simulink model "ofdm_txrx_mimo"  (Simulink 7.1)
settings: Solver=ode45  StartTime=0.0  StopTime=6e3  RelTol=1e-3
PreLoadFcn:
  | ofdm_rx_mimo_init
InitFcn:
  | ofdm_rx_mimo_init
StartFcn:
  | ofdm_rx_mimo_init
values from neighbouring files (symbols this model uses):
  BER_RAM_init_size = 2048    [ofdm_tx_mimo_init.m]
  CPLength = 16    [ofdm_tx_mimo_init.m]
  CRCPolynomial = hex2dec('04c11db7')    [ofdm_tx_mimo_init.m]
  freqOffset_track_filtCoef_i = hex2dec('2500')/2^32    [ofdm_rx_mimo_init.m]
  freqOffset_track_filtCoef_p = hex2dec('d0000')/2^32    [ofdm_rx_mimo_init.m]
  hi = zeros(1,length(cci))    [ofdm_rx_mimo_init.m]
  hr = zeros(1,length(ccr))    [ofdm_rx_mimo_init.m]
  max_CP_length = 16    [ofdm_tx_mimo_init.m]
  max_num_baseRateSymbols = 31    [ofdm_tx_mimo_init.m]
  max_num_subcarriers = 64    [ofdm_tx_mimo_init.m]
  max_num_trainingSymbols = 15    [ofdm_tx_mimo_init.m]
  max_numBytes = 16384    [ofdm_tx_mimo_init.m]
  max_OFDM_symbols = 2047    [ofdm_tx_mimo_init.m]
  modConstellation_qam16 = .75*[1 3 -1 -3]./3    [ofdm_tx_mimo_init.m]
  modConstellation_qam256 = 0.9375*[3 1 5 7 11 9 13 15 -3 -1 -5 -7 -11 -9 -13 -15]./15    [ofdm_tx_mimo_init.m]
  modConstellation_qam64 = 0.875*[3 1 5 7 -3 -1 -5 -7]./7    [ofdm_tx_mimo_init.m]
  modConstellation_qpsk = [1 -1]./sqrt(2)    [ofdm_tx_mimo_init.m]
  numBaseRateSymbols = 2    [ofdm_tx_mimo_init.m]
  numSubcarriers = 64    [ofdm_tx_mimo_init.m]
  numTrainingSymbols = 2    [ofdm_tx_mimo_init.m]
  pilot1_indicies = 7 + ( (64-7) * 2^16)    (= 3735559)    [ofdm_tx_mimo_init.m]
  pilot2_indicies = 21 + ( (64-21) * 2^16)    (= 2818069)    [ofdm_tx_mimo_init.m]
  pilotValue_neg = hex2dec('8000') + (2^16 * 0)    [ofdm_tx_mimo_init.m]
  pilotValue_pos = hex2dec('7FFF') + (2^16 * 0)    [ofdm_tx_mimo_init.m]
  pkt_crossCorr_thresh = 0.7    [ofdm_rx_mimo_init.m]
  pkt_energy_thresh = 0    [ofdm_rx_mimo_init.m]
  pkt_numPayloadBytes = 1400+42-6    (= 1436)    [ofdm_tx_mimo_init.m]
  pktByteNums = numHeaderBytes +  				byteIndex_numPayloadBytes(1)*2^8 +  				byteIndex_numPayloadBytes(2)*2^16 +  				byteIndex_simpleDynModMasks * 2^24    [ofdm_rx_mimo_init.m]
  PktDet_Delay = 26 + Symbol_Timing_Offset*2^7    (= 1050)    [ofdm_rx_mimo_init.m]
  preamble = 6*[0 shortsyms_10 longsyms_2]    [ofdm_tx_mimo_init.m]
  preamble_I = real(preamble)    [ofdm_tx_mimo_init.m]
  preamble_Q = imag(preamble)    [ofdm_tx_mimo_init.m]
  RAM_init_size = max_numBytes    (= 16384)    [ofdm_tx_mimo_init.m]
  reg_Interrupt_PktBuf_Control = reg_InterruptControl +  	1 * 2^16 +  	0 * 2^24    (= 65656)    [ofdm_rx_mimo_init.m]
  rx_controlBits = 1 * 2  		 + 1 * 4  		 + 0 * 8  		 + 1 * 16 * rx_SISO_Mode  		 + 1 * 32  		 + 1 * 64  		 + 0 * 128  		 + 1 * 256  		 + 0 * 512  		 + 1 * 1024  		 + 0 * 2048  		 + 1 * 4096  		 + 1 * 8192  		 + 0 * 16384  		 + 1 * 65536  		 + 1 * 131072  		 + 0 * 262144  		 + 1 * 524288    [ofdm_rx_mimo_init.m]
  Rx_PhaseNoiseTrack_K = 0.6094    [ofdm_rx_mimo_init.m]
  rx_postEq_scaling = round(rxScaling*2^11) * (1 + 2^16)    [ofdm_rx_mimo_init.m]
  RxDataScrambling_Seq = [40 198 78 63 82 173 102 245 48 111 172 115 147 230 216 93 72 65 62 2 205 242 122 90 128 83 105 97 73 10 5 252]    [ofdm_tx_mimo_init.m]
  simOnly_numSamples = length(preamble)+( (numSubcarriers+CPLength)*(numTrainingSymbols + numBaseRateSymbols + numFullRateSymbols/2) )    [ofdm_tx_mimo_init.m]
  train = [train train]    [ofdm_tx_mimo_init.m]
  tx_controlBits = (antB_preambleShift * 2^4) + (2^0 * tx_SISO_Mode) + 4*0 + 8*1    (= 73)    [ofdm_tx_mimo_init.m]
  TxDataScrambling_Seq = [40 198 78 63 82 173 102 245 48 111 172 115 147 230 216 93 72 65 62 2 205 242 122 90 128 83 105 97 73 10 5 252]    [ofdm_tx_mimo_init.m]
  txReg_symbolCounts = (2^16 * numFullRateSymbols) + (2^8 *numBaseRateSymbols ) + numTrainingSymbols    [ofdm_tx_mimo_init.m]
  TxRx_FFTScaling = bin2dec('010111') + (bin2dec('000101') * 2^6)    [ofdm_tx_mimo_init.m]
undefined symbols (not defined in the file or in its folder): AntA, AntB, BaseRate, CRC_table_gen, gcbh, intfilt, pktTiming_count_payloadStart, pktTiming_symbol_length, xlBlockLoadCallback, xlGetSimulinkPeriod
libraries: xbsIndex_r4 x4212, dspmlti4 x4, dspsigops x4, commchan3 x2, simulink x2, commrflib2 x2, dspsrcs4 x1
(part 8 of 43)

== system ofdm_txrx_mimo/Memory-mapped Registers/Rx Registers/Read-Write Register2 ==
  Constant : xbsIndex_r4/Constant  arith_type=Boolean  const=1  n_bits=16  bin_pt=14  explicit_period=on  period=sampPeriod  equ=P=C  opselect=C  inp2=PCIN>>17  opr=+  inp1=P  carry=CIN  dbl_ovrd=off  block_type=constant
      settings: block_version=8.2.02
  From Register : xbsIndex_r4/From Register  shared_memory_name='Rx_pktByteNums_w'  init=131864  period=sampPeriod  ownership=Locally owned and initialized  arith_type=Unsigned  n_bits=32  bin_pt=0  dbl_ovrd=off  block_type=fromreg
      settings: AttributesFormatString=From Register\n<< %<shared_memory_name> >>\n%<n_bits>\n%<period>\n%<bin_pt>\n%<arith_type>\n%<ownership>\n%<init>  infoedit="Register block that reads data to a shared memory register.  Delay of one sample period."  block_version=8.2.02
  Terminator : Terminator
  To Register : xbsIndex_r4/To Register  shared_memory_name='Rx_pktByteNums_r'  init=131864  ownership=Locally owned and initialized  explicit_data_type=on  arith_type=Unsigned  n_bits=32  bin_pt=0  dbl_ovrd=off  block_type=toreg
      settings: AttributesFormatString=To Register\n<< %<shared_memory_name> >>  infoedit="Register block that writes data to a shared memory register.  Delay of one sample period."  block_version=8.2.02
  D : Outport  OutDataType=sfix(16)  OutScaling=2^0
  wires:
  To Register/1 -> Terminator/1
  Constant/1 -> To Register/2
  From Register/1 -> To Register/1, D/1

== system ofdm_txrx_mimo/Memory-mapped Registers/Rx Registers/Read-Write Register3 ==
  Constant : xbsIndex_r4/Constant  arith_type=Boolean  const=1  n_bits=16  bin_pt=14  explicit_period=on  period=sampPeriod  equ=P=C  opselect=C  inp2=PCIN>>17  opr=+  inp1=P  carry=CIN  dbl_ovrd=off  block_type=constant
      settings: block_version=8.2.02
  From Register : xbsIndex_r4/From Register  shared_memory_name='Rx_PktDet_Delay_w'  init=1050  period=sampPeriod  ownership=Locally owned and initialized  arith_type=Unsigned  n_bits=32  bin_pt=0  dbl_ovrd=off  block_type=fromreg
      settings: AttributesFormatString=From Register\n<< %<shared_memory_name> >>\n%<n_bits>\n%<period>\n%<bin_pt>\n%<arith_type>\n%<ownership>\n%<init>  infoedit="Register block that reads data to a shared memory register.  Delay of one sample period."  block_version=8.2.02
  Terminator : Terminator
  To Register : xbsIndex_r4/To Register  shared_memory_name='Rx_PktDet_Delay_r'  init=1050  ownership=Locally owned and initialized  explicit_data_type=on  arith_type=Unsigned  n_bits=32  bin_pt=0  dbl_ovrd=off  block_type=toreg
      settings: AttributesFormatString=To Register\n<< %<shared_memory_name> >>  infoedit="Register block that writes data to a shared memory register.  Delay of one sample period."  block_version=8.2.02
  D : Outport  OutDataType=sfix(16)  OutScaling=2^0
  wires:
  To Register/1 -> Terminator/1
  Constant/1 -> To Register/2
  From Register/1 -> To Register/1, D/1

== system ofdm_txrx_mimo/Memory-mapped Registers/Rx Registers/Read-Write Register4 ==
  Constant : xbsIndex_r4/Constant  arith_type=Boolean  const=1  n_bits=16  bin_pt=14  explicit_period=on  period=sampPeriod  equ=P=C  opselect=C  inp2=PCIN>>17  opr=+  inp1=P  carry=CIN  dbl_ovrd=off  block_type=constant
      settings: block_version=8.2.02
  From Register : xbsIndex_r4/From Register  shared_memory_name='Rx_OFDM_SymbolCounts_w'  init=131074  period=sampPeriod  ownership=Locally owned and initialized  arith_type=Unsigned  n_bits=32  bin_pt=0  dbl_ovrd=off  block_type=fromreg
      settings: AttributesFormatString=From Register\n<< %<shared_memory_name> >>\n%<n_bits>\n%<period>\n%<bin_pt>\n%<arith_type>\n%<ownership>\n%<init>  infoedit="Register block that reads data to a shared memory register.  Delay of one sample period."  block_version=8.2.02
  Terminator : Terminator
  To Register : xbsIndex_r4/To Register  shared_memory_name='Rx_OFDM_SymbolCounts_r'  init=131074  ownership=Locally owned and initialized  explicit_data_type=on  arith_type=Unsigned  n_bits=32  bin_pt=0  dbl_ovrd=off  block_type=toreg
      settings: AttributesFormatString=To Register\n<< %<shared_memory_name> >>  infoedit="Register block that writes data to a shared memory register.  Delay of one sample period."  block_version=8.2.02
  D : Outport  OutDataType=sfix(16)  OutScaling=2^0
  wires:
  To Register/1 -> Terminator/1
  Constant/1 -> To Register/2
  From Register/1 -> To Register/1, D/1

== system ofdm_txrx_mimo/Memory-mapped Registers/Rx Registers/Read-Write Register5 ==
  Constant : xbsIndex_r4/Constant  arith_type=Boolean  const=1  n_bits=16  bin_pt=14  explicit_period=on  period=sampPeriod  equ=P=C  opselect=C  inp2=PCIN>>17  opr=+  inp1=P  carry=CIN  dbl_ovrd=off  block_type=constant
      settings: block_version=8.2.02
  From Register : xbsIndex_r4/From Register  shared_memory_name='Rx_ControlBits_w'  init=734582  period=sampPeriod  ownership=Locally owned and initialized  arith_type=Unsigned  n_bits=32  bin_pt=0  dbl_ovrd=off  block_type=fromreg
      settings: AttributesFormatString=From Register\n<< %<shared_memory_name> >>\n%<n_bits>\n%<period>\n%<bin_pt>\n%<arith_type>\n%<ownership>\n%<init>  infoedit="Register block that reads data to a shared memory register.  Delay of one sample period."  block_version=8.2.02
  Terminator : Terminator
  To Register : xbsIndex_r4/To Register  shared_memory_name='Rx_ControlBits_r'  init=734582  ownership=Locally owned and initialized  explicit_data_type=on  arith_type=Unsigned  n_bits=32  bin_pt=0  dbl_ovrd=off  block_type=toreg
      settings: AttributesFormatString=To Register\n<< %<shared_memory_name> >>  infoedit="Register block that writes data to a shared memory register.  Delay of one sample period."  block_version=8.2.02
  D : Outport  OutDataType=sfix(16)  OutScaling=2^0
  wires:
  From Register/1 -> D/1, To Register/1
  Constant/1 -> To Register/2
  To Register/1 -> Terminator/1

== system ofdm_txrx_mimo/Memory-mapped Registers/Rx Registers/Write-Only Register ==
  From Register : xbsIndex_r4/From Register  shared_memory_name='Rx_pktDet_Tresholds_w'  init=0.7  period=sampPeriod  ownership=Locally owned and initialized  arith_type=Unsigned  n_bits=32  bin_pt=0  dbl_ovrd=off  block_type=fromreg
      settings: AttributesFormatString=From Register\n<< %<shared_memory_name> >>\n%<n_bits>\n%<period>\n%<bin_pt>\n%<arith_type>\n%<ownership>\n%<init>  infoedit="Register block that reads data to a shared memory register.  Delay of one sample period."  block_version=8.2.02
  D : Outport  OutDataType=sfix(16)  OutScaling=2^0
  wires:
  From Register/1 -> D/1

== system ofdm_txrx_mimo/Memory-mapped Registers/Rx Registers/Write-Only Register1 ==
  From Register : xbsIndex_r4/From Register  shared_memory_name='Rx_Constellation_Scaling_w'  init=268439552  period=sampPeriod  ownership=Locally owned and initialized  arith_type=Unsigned  n_bits=32  bin_pt=0  dbl_ovrd=off  block_type=fromreg
      settings: AttributesFormatString=From Register\n<< %<shared_memory_name> >>\n%<n_bits>\n%<period>\n%<bin_pt>\n%<arith_type>\n%<ownership>\n%<init>  infoedit="Register block that reads data to a shared memory register.  Delay of one sample period."  block_version=8.2.02
  D : Outport  OutDataType=sfix(16)  OutScaling=2^0
  wires:
  From Register/1 -> D/1

== system ofdm_txrx_mimo/Memory-mapped Registers/Rx Registers/Write-Only Register2 ==
  From Register : xbsIndex_r4/From Register  shared_memory_name='Rx_FreqOffFilt_B_KI_w'  init=9472  period=sampPeriod  ownership=Locally owned and initialized  arith_type=Unsigned  n_bits=32  bin_pt=0  dbl_ovrd=off  block_type=fromreg
      settings: AttributesFormatString=From Register\n<< %<shared_memory_name> >>\n%<n_bits>\n%<period>\n%<bin_pt>\n%<arith_type>\n%<ownership>\n%<init>  infoedit="Register block that reads data to a shared memory register.  Delay of one sample period."  block_version=8.2.02
  D : Outport  OutDataType=sfix(16)  OutScaling=2^0
  wires:
  From Register/1 -> D/1

== system ofdm_txrx_mimo/Memory-mapped Registers/Rx Registers/Write-Only Register3 ==
  From Register : xbsIndex_r4/From Register  shared_memory_name='Rx_FreqOffFilt_B_KP_w'  init=851968  period=sampPeriod  ownership=Locally owned and initialized  arith_type=Unsigned  n_bits=32  bin_pt=0  dbl_ovrd=off  block_type=fromreg
      settings: AttributesFormatString=From Register\n<< %<shared_memory_name> >>\n%<n_bits>\n%<period>\n%<bin_pt>\n%<arith_type>\n%<ownership>\n%<init>  infoedit="Register block that reads data to a shared memory register.  Delay of one sample period."  block_version=8.2.02
  D : Outport  OutDataType=sfix(16)  OutScaling=2^0
  wires:
  From Register/1 -> D/1

== system ofdm_txrx_mimo/Memory-mapped Registers/Rx Registers/Write-Only Register4 ==
  From Register : xbsIndex_r4/From Register  shared_memory_name='Rx_PhaseNoiseTrack_K_w'  init=40896141.7216  period=sampPeriod  ownership=Locally owned and initialized  arith_type=Unsigned  n_bits=32  bin_pt=0  dbl_ovrd=off  block_type=fromreg
      settings: AttributesFormatString=From Register\n<< %<shared_memory_name> >>\n%<n_bits>\n%<period>\n%<bin_pt>\n%<arith_type>\n%<ownership>\n%<init>  infoedit="Register block that reads data to a shared memory register.  Delay of one sample period."  block_version=8.2.02
  D : Outport  OutDataType=sfix(16)  OutScaling=2^0
  wires:
  From Register/1 -> D/1

== system ofdm_txrx_mimo/Memory-mapped Registers/Rx Registers/Write-Only Register5 ==
  From Register : xbsIndex_r4/From Register  shared_memory_name='Rx_EVM_w'  init=20  period=sampPeriod  ownership=Locally owned and initialized  arith_type=Unsigned  n_bits=7  bin_pt=0  dbl_ovrd=off  block_type=fromreg
      settings: AttributesFormatString=From Register\n<< %<shared_memory_name> >>\n%<n_bits>\n%<period>\n%<bin_pt>\n%<arith_type>\n%<ownership>\n%<init>  infoedit="Register block that reads data to a shared memory register.  Delay of one sample period."  block_version=8.2.02
  D : Outport  OutDataType=sfix(16)  OutScaling=2^0
  wires:
  From Register/1 -> D/1

== system ofdm_txrx_mimo/Memory-mapped Registers/Tx Registers/Read Only Register ==
  D : Inport  OutDataType=sfix(16)  OutScaling=2^0
  En : Inport  Port=2  OutDataType=sfix(16)  OutScaling=2^0
  Convert : xbsIndex_r4/Convert  arith_type=Unsigned  n_bits=32  bin_pt=0  quantization=Truncate  overflow=Wrap  latency=0  dbl_ovrd=off  pipeline=off  block_type=convert
      settings: block_version=10.1
  Reinterpret : xbsIndex_r4/Reinterpret  force_arith_type=on  arith_type=Unsigned  force_bin_pt=on  bin_pt=0  block_type=reinterpret
      settings: infoedit="Changes signal type without altering the binary representation.   You can changed the signal between signed and unsigned, and relocate the binary point.<P><P>Hardware notes: In hardware this block costs nothing.<P><P>Example:  Suppose the input is 6 bits wide, signed, with 2 fractional bits, and the output is forced to unsigned with 0 fractional bits.  Then an input of -2.0 (1110.00 in binary 2's complement) becomes an output of 56 (111000 in binary)."  block_version=10.1
  Terminator : Terminator
  To Register : xbsIndex_r4/To Register  shared_memory_name='Tx_PktRunning'  init=0  ownership=Locally owned and initialized  explicit_data_type=on  arith_type=Unsigned  n_bits=32  bin_pt=0  dbl_ovrd=off  block_type=toreg
      settings: AttributesFormatString=To Register\n<< %<shared_memory_name> >>  infoedit="Register block that writes data to a shared memory register.  Delay of one sample period."  block_version=8.2.02
  wires:
  En/1 -> To Register/2
  To Register/1 -> Terminator/1
  D/1 -> Reinterpret/1
  Reinterpret/1 -> Convert/1
  Convert/1 -> To Register/1

== system ofdm_txrx_mimo/Memory-mapped Registers/Tx Registers/Read-Write Register ==
  Constant : xbsIndex_r4/Constant  arith_type=Boolean  const=1  n_bits=16  bin_pt=14  explicit_period=on  period=sampPeriod  equ=P=C  opselect=C  inp2=PCIN>>17  opr=+  inp1=P  carry=CIN  dbl_ovrd=off  block_type=constant
      settings: block_version=8.2.02
  From Register : xbsIndex_r4/From Register  shared_memory_name='Tx_Start_Reset_Control_w'  init=0  period=sampPeriod  ownership=Locally owned and initialized  arith_type=Unsigned  n_bits=32  bin_pt=0  dbl_ovrd=off  block_type=fromreg
      settings: AttributesFormatString=From Register\n<< %<shared_memory_name> >>\n%<n_bits>\n%<period>\n%<bin_pt>\n%<arith_type>\n%<ownership>\n%<init>  infoedit="Register block that reads data to a shared memory register.  Delay of one sample period."  block_version=8.2.02
  Terminator : Terminator
  To Register : xbsIndex_r4/To Register  shared_memory_name='Tx_Start_Reset_Control_r'  init=0  ownership=Locally owned and initialized  explicit_data_type=on  arith_type=Unsigned  n_bits=32  bin_pt=0  dbl_ovrd=off  block_type=toreg
      settings: AttributesFormatString=To Register\n<< %<shared_memory_name> >>  infoedit="Register block that writes data to a shared memory register.  Delay of one sample period."  block_version=8.2.02
  D : Outport  OutDataType=sfix(16)  OutScaling=2^0
  wires:
  To Register/1 -> Terminator/1
  Constant/1 -> To Register/2
  From Register/1 -> To Register/1, D/1

== system ofdm_txrx_mimo/Memory-mapped Registers/Tx Registers/Read-Write Register1 ==
  Constant : xbsIndex_r4/Constant  arith_type=Boolean  const=1  n_bits=16  bin_pt=14  explicit_period=on  period=sampPeriod  equ=P=C  opselect=C  inp2=PCIN>>17  opr=+  inp1=P  carry=CIN  dbl_ovrd=off  block_type=constant
      settings: block_version=8.2.02
  From Register : xbsIndex_r4/From Register  shared_memory_name='Tx_ControlBits_w'  init=73  period=sampPeriod  ownership=Locally owned and initialized  arith_type=Unsigned  n_bits=32  bin_pt=0  dbl_ovrd=off  block_type=fromreg
      settings: AttributesFormatString=From Register\n<< %<shared_memory_name> >>\n%<n_bits>\n%<period>\n%<bin_pt>\n%<arith_type>\n%<ownership>\n%<init>  infoedit="Register block that reads data to a shared memory register.  Delay of one sample period."  block_version=8.2.02
  Terminator : Terminator
  To Register : xbsIndex_r4/To Register  shared_memory_name='Tx_ControlBits_r'  init=73  ownership=Locally owned and initialized  explicit_data_type=on  arith_type=Unsigned  n_bits=32  bin_pt=0  dbl_ovrd=off  block_type=toreg
      settings: AttributesFormatString=To Register\n<< %<shared_memory_name> >>  infoedit="Register block that writes data to a shared memory register.  Delay of one sample period."  block_version=8.2.02
  D : Outport  OutDataType=sfix(16)  OutScaling=2^0
  wires:
  To Register/1 -> Terminator/1
  Constant/1 -> To Register/2
  From Register/1 -> To Register/1, D/1

== system ofdm_txrx_mimo/Memory-mapped Registers/Tx Registers/Read-Write Register2 ==
  Constant : xbsIndex_r4/Constant  arith_type=Boolean  const=1  n_bits=16  bin_pt=14  explicit_period=on  period=sampPeriod  equ=P=C  opselect=C  inp2=PCIN>>17  opr=+  inp1=P  carry=CIN  dbl_ovrd=off  block_type=constant
      settings: block_version=8.2.02
  From Register : xbsIndex_r4/From Register  shared_memory_name='Tx_OFDM_SymCounts_w'  init=15729154  period=sampPeriod  ownership=Locally owned and initialized  arith_type=Unsigned  n_bits=32  bin_pt=0  dbl_ovrd=off  block_type=fromreg
      settings: AttributesFormatString=From Register\n<< %<shared_memory_name> >>\n%<n_bits>\n%<period>\n%<bin_pt>\n%<arith_type>\n%<ownership>\n%<init>  infoedit="Register block that reads data to a shared memory register.  Delay of one sample period."  block_version=8.2.02
  Terminator : Terminator
  To Register : xbsIndex_r4/To Register  shared_memory_name='Tx_OFDM_SymCounts_r'  init=15729154  ownership=Locally owned and initialized  explicit_data_type=on  arith_type=Unsigned  n_bits=32  bin_pt=0  dbl_ovrd=off  block_type=toreg
      settings: AttributesFormatString=To Register\n<< %<shared_memory_name> >>  infoedit="Register block that writes data to a shared memory register.  Delay of one sample period."  block_version=8.2.02
  D : Outport  OutDataType=sfix(16)  OutScaling=2^0
  wires:
  To Register/1 -> Terminator/1
  Constant/1 -> To Register/2
  From Register/1 -> To Register/1, D/1

== system ofdm_txrx_mimo/Memory-mapped Registers/Tx Registers/Write-Only Register ==
  From Register : xbsIndex_r4/From Register  shared_memory_name='Tx_PreambleMasking_w'  init=0  period=sampPeriod  ownership=Locally owned and initialized  arith_type=Unsigned  n_bits=32  bin_pt=0  dbl_ovrd=off  block_type=fromreg
      settings: AttributesFormatString=From Register\n<< %<shared_memory_name> >>\n%<n_bits>\n%<period>\n%<bin_pt>\n%<arith_type>\n%<ownership>\n%<init>  infoedit="Register block that reads data to a shared memory register.  Delay of one sample period."  block_version=8.2.02
  D : Outport  OutDataType=sfix(16)  OutScaling=2^0
  wires:
  From Register/1 -> D/1

== system ofdm_txrx_mimo/Memory-mapped Registers/Tx Registers/Write-Only Register1 ==
  From Register : xbsIndex_r4/From Register  shared_memory_name='Tx_Scaling_w'  init=1768954592  period=sampPeriod  ownership=Locally owned and initialized  arith_type=Unsigned  n_bits=32  bin_pt=0  dbl_ovrd=off  block_type=fromreg
      settings: AttributesFormatString=From Register\n<< %<shared_memory_name> >>\n%<n_bits>\n%<period>\n%<bin_pt>\n%<arith_type>\n%<ownership>\n%<init>  infoedit="Register block that reads data to a shared memory register.  Delay of one sample period."  block_version=8.2.02
  D : Outport  OutDataType=sfix(16)  OutScaling=2^0
  wires:
  From Register/1 -> D/1

== system ofdm_txrx_mimo/Memory-mapped Registers/Tx Registers/Write-Only Register2 ==
  From Register : xbsIndex_r4/From Register  shared_memory_name='Tx_Pilots_Index1_w'  init=3735559  period=sampPeriod  ownership=Locally owned and initialized  arith_type=Unsigned  n_bits=32  bin_pt=0  dbl_ovrd=off  block_type=fromreg
      settings: AttributesFormatString=From Register\n<< %<shared_memory_name> >>\n%<n_bits>\n%<period>\n%<bin_pt>\n%<arith_type>\n%<ownership>\n%<init>  infoedit="Register block that reads data to a shared memory register.  Delay of one sample period."  block_version=8.2.02
  D : Outport  OutDataType=sfix(16)  OutScaling=2^0
  wires:
  From Register/1 -> D/1

== system ofdm_txrx_mimo/Memory-mapped Registers/Tx Registers/Write-Only Register3 ==
  From Register : xbsIndex_r4/From Register  shared_memory_name='Tx_Pilots_Index2_w'  init=2818069  period=sampPeriod  ownership=Locally owned and initialized  arith_type=Unsigned  n_bits=32  bin_pt=0  dbl_ovrd=off  block_type=fromreg
      settings: AttributesFormatString=From Register\n<< %<shared_memory_name> >>\n%<n_bits>\n%<period>\n%<bin_pt>\n%<arith_type>\n%<ownership>\n%<init>  infoedit="Register block that reads data to a shared memory register.  Delay of one sample period."  block_version=8.2.02
  D : Outport  OutDataType=sfix(16)  OutScaling=2^0
  wires:
  From Register/1 -> D/1

== system ofdm_txrx_mimo/Memory-mapped Registers/Tx Registers/Write-Only Register4 ==
  From Register : xbsIndex_r4/From Register  shared_memory_name='Tx_Pilots_Value1_w'  init=32768  period=sampPeriod  ownership=Locally owned and initialized  arith_type=Unsigned  n_bits=32  bin_pt=0  dbl_ovrd=off  block_type=fromreg
      settings: AttributesFormatString=From Register\n<< %<shared_memory_name> >>\n%<n_bits>\n%<period>\n%<bin_pt>\n%<arith_type>\n%<ownership>\n%<init>  infoedit="Register block that reads data to a shared memory register.  Delay of one sample period."  block_version=8.2.02
  D : Outport  OutDataType=sfix(16)  OutScaling=2^0
  wires:
  From Register/1 -> D/1

== system ofdm_txrx_mimo/Memory-mapped Registers/Tx Registers/Write-Only Register5 ==
  From Register : xbsIndex_r4/From Register  shared_memory_name='Tx_Pilots_Value2_w'  init=32767  period=sampPeriod  ownership=Locally owned and initialized  arith_type=Unsigned  n_bits=32  bin_pt=0  dbl_ovrd=off  block_type=fromreg
      settings: AttributesFormatString=From Register\n<< %<shared_memory_name> >>\n%<n_bits>\n%<period>\n%<bin_pt>\n%<arith_type>\n%<ownership>\n%<init>  infoedit="Register block that reads data to a shared memory register.  Delay of one sample period."  block_version=8.2.02
  D : Outport  OutDataType=sfix(16)  OutScaling=2^0
  wires:
  From Register/1 -> D/1

== system ofdm_txrx_mimo/Memory-mapped Registers/TxRx Registers/InterruptControl ==
  Reg_8b : Inport  OutDataType=sfix(16)  OutScaling=2^0
  LSB+0 : xbsIndex_r4/Slice  nbits=1  boolean_output=on  mode=Lower Bit Location + Width  bit1=0  base1=MSB of Input  bit0=0  base0=LSB of Input  dbl_ovrd=off  block_type=slice
      settings: infoedit="Extracts a given range of bits from each input sample and presents it at the output.  The output type is ordinarily unsigned with binary point at zero, but can be Boolean when the slice is one bit wide.<P><P>Hardware notes: In hardware this block costs nothing."  block_version=VER_STRING_GOES_HERE
  LSB+1 : xbsIndex_r4/Slice  nbits=1  boolean_output=on  mode=Lower Bit Location + Width  bit1=0  base1=MSB of Input  bit0=1  base0=LSB of Input  dbl_ovrd=off  block_type=slice
      settings: infoedit="Extracts a given range of bits from each input sample and presents it at the output.  The output type is ordinarily unsigned with binary point at zero, but can be Boolean when the slice is one bit wide.<P><P>Hardware notes: In hardware this block costs nothing."  block_version=VER_STRING_GOES_HERE
  LSB+2 : xbsIndex_r4/Slice  nbits=1  boolean_output=off  mode=Lower Bit Location + Width  bit1=0  base1=MSB of Input  bit0=2  base0=LSB of Input  dbl_ovrd=off  block_type=slice
      settings: infoedit="Extracts a given range of bits from each input sample and presents it at the output.  The output type is ordinarily unsigned with binary point at zero, but can be Boolean when the slice is one bit wide.<P><P>Hardware notes: In hardware this block costs nothing."  block_version=VER_STRING_GOES_HERE
  LSB+3 : xbsIndex_r4/Slice  nbits=1  boolean_output=off  mode=Lower Bit Location + Width  bit1=0  base1=MSB of Input  bit0=3  base0=LSB of Input  dbl_ovrd=off  block_type=slice
      settings: infoedit="Extracts a given range of bits from each input sample and presents it at the output.  The output type is ordinarily unsigned with binary point at zero, but can be Boolean when the slice is one bit wide.<P><P>Hardware notes: In hardware this block costs nothing."  block_version=VER_STRING_GOES_HERE
  LSB+4 : xbsIndex_r4/Slice  nbits=1  boolean_output=off  mode=Lower Bit Location + Width  bit1=0  base1=MSB of Input  bit0=4  base0=LSB of Input  dbl_ovrd=off  block_type=slice
      settings: infoedit="Extracts a given range of bits from each input sample and presents it at the output.  The output type is ordinarily unsigned with binary point at zero, but can be Boolean when the slice is one bit wide.<P><P>Hardware notes: In hardware this block costs nothing."  block_version=VER_STRING_GOES_HERE
  LSB+5 : xbsIndex_r4/Slice  nbits=1  boolean_output=off  mode=Lower Bit Location + Width  bit1=0  base1=MSB of Input  bit0=5  base0=LSB of Input  dbl_ovrd=off  block_type=slice
      settings: infoedit="Extracts a given range of bits from each input sample and presents it at the output.  The output type is ordinarily unsigned with binary point at zero, but can be Boolean when the slice is one bit wide.<P><P>Hardware notes: In hardware this block costs nothing."  block_version=VER_STRING_GOES_HERE
  LSB+6 : xbsIndex_r4/Slice  nbits=1  boolean_output=off  mode=Lower Bit Location + Width  bit1=0  base1=MSB of Input  bit0=6  base0=LSB of Input  dbl_ovrd=off  block_type=slice
      settings: infoedit="Extracts a given range of bits from each input sample and presents it at the output.  The output type is ordinarily unsigned with binary point at zero, but can be Boolean when the slice is one bit wide.<P><P>Hardware notes: In hardware this block costs nothing."  block_version=VER_STRING_GOES_HERE
  LSB+7 : xbsIndex_r4/Slice  nbits=1  boolean_output=off  mode=Lower Bit Location + Width  bit1=0  base1=MSB of Input  bit0=7  base0=LSB of Input  dbl_ovrd=off  block_type=slice
      settings: infoedit="Extracts a given range of bits from each input sample and presents it at the output.  The output type is ordinarily unsigned with binary point at zero, but can be Boolean when the slice is one bit wide.<P><P>Hardware notes: In hardware this block costs nothing."  block_version=VER_STRING_GOES_HERE
  Posedge : Subsystem
  Posedge1 : Subsystem
  Posedge2 : Subsystem
  tag scope: intrCtrl_RxBadHeader_en=global, intrCtrl_RxBadPkt_en=global, intrCtrl_RxGoodHeader_en=global, intrCtrl_RxGoodPkt_en=global, intrCtrl_RxHeaderInterrupts_rst=global, intrCtrl_RxPktInterrupts_rst=global, intrCtrl_TxDone_en=global, intrCtrl_TxDone_rst=global
  wires:
  LSB+0/1 -> Posedge/1(D)
  LSB+1/1 -> Posedge1/1(D)
  Reg_8b/1 -> LSB+0/1, LSB+1/1, LSB+2/1, LSB+4/1, LSB+5/1, LSB+6/1, LSB+7/1, LSB+3/1
  Posedge/1(Q) -> [intrCtrl_RxPktInterrupts_rst]
  Posedge1/1(Q) -> [intrCtrl_RxHeaderInterrupts_rst]
  LSB+4/1 -> [intrCtrl_RxBadPkt_en]
  LSB+5/1 -> [intrCtrl_RxGoodHeader_en]
  LSB+6/1 -> [intrCtrl_RxBadHeader_en]
  LSB+7/1 -> [intrCtrl_TxDone_en]
  LSB+3/1 -> [intrCtrl_RxGoodPkt_en]
  LSB+2/1 -> Posedge2/1(D)
  Posedge2/1(Q) -> [intrCtrl_TxDone_rst]

== system ofdm_txrx_mimo/Memory-mapped Registers/TxRx Registers/Read-Write Register ==
  Constant : xbsIndex_r4/Constant  arith_type=Boolean  const=1  n_bits=16  bin_pt=14  explicit_period=on  period=sampPeriod  equ=P=C  opselect=C  inp2=PCIN>>17  opr=+  inp1=P  carry=CIN  dbl_ovrd=off  block_type=constant
      settings: block_version=8.2.02
  From Register : xbsIndex_r4/From Register  shared_memory_name='TxRx_Interrupt_PktBuf_Ctrl_w'  init=65656  period=sampPeriod  ownership=Locally owned and initialized  arith_type=Unsigned  n_bits=32  bin_pt=0  dbl_ovrd=off  block_type=fromreg
      settings: AttributesFormatString=From Register\n<< %<shared_memory_name> >>\n%<n_bits>\n%<period>\n%<bin_pt>\n%<arith_type>\n%<ownership>\n%<init>  infoedit="Register block that reads data to a shared memory register.  Delay of one sample period."  block_version=8.2.02
  Terminator : Terminator
  To Register : xbsIndex_r4/To Register  shared_memory_name='TxRx_Interrupt_PktBuf_Ctrl_r'  init=65656  ownership=Locally owned and initialized  explicit_data_type=on  arith_type=Unsigned  n_bits=32  bin_pt=0  dbl_ovrd=off  block_type=toreg
      settings: AttributesFormatString=To Register\n<< %<shared_memory_name> >>  infoedit="Register block that writes data to a shared memory register.  Delay of one sample period."  block_version=8.2.02
  D : Outport  OutDataType=sfix(16)  OutScaling=2^0
  wires:
  To Register/1 -> Terminator/1
  Constant/1 -> To Register/2
  From Register/1 -> To Register/1, D/1

== system ofdm_txrx_mimo/Memory-mapped Registers/TxRx Registers/Write-Only Register ==
  From Register : xbsIndex_r4/From Register  shared_memory_name='TxRx_FFT_Scaling_w'  init=343  period=sampPeriod  ownership=Locally owned and initialized  arith_type=Unsigned  n_bits=12  bin_pt=0  dbl_ovrd=off  block_type=fromreg
      settings: AttributesFormatString=From Register\n<< %<shared_memory_name> >>\n%<n_bits>\n%<period>\n%<bin_pt>\n%<arith_type>\n%<ownership>\n%<init>  infoedit="Register block that reads data to a shared memory register.  Delay of one sample period."  block_version=8.2.02
  D : Outport  OutDataType=sfix(16)  OutScaling=2^0
  wires:
  From Register/1 -> D/1

== system ofdm_txrx_mimo/OFDM Rx MIMO/ADC Inputs & Antenna Selection/Antenna Selection ==
  AntA_I : Inport  OutDataType=sfix(16)  OutScaling=2^0
  AntA_Q : Inport  Port=2  OutDataType=sfix(16)  OutScaling=2^0
  AntB_I : Inport  Port=3  OutDataType=sfix(16)  OutScaling=2^0
  AntB_Q : Inport  Port=4  OutDataType=sfix(16)  OutScaling=2^0
  PktDetReset : Inport  Port=5  OutDataType=sfix(16)  OutScaling=2^0
  Ant Sel Logic : Subsystem
  AntA_Gains : Subsystem
  AntB_Gains : Subsystem
  Concat2 : xbsIndex_r4/Concat  num_inputs=3  dbl_ovrd=off  block_type=concat
      settings: block_version=VER_STRING_GOES_HERE
  Constant6 : xbsIndex_r4/Constant  arith_type=Unsigned  const=0  n_bits=1  bin_pt=0  explicit_period=off  period=1  equ=P=C  opselect=C  inp2=PCIN>>17  opr=+  inp1=P  carry=CIN  dbl_ovrd=off  block_type=constant
      settings: block_version=VER_STRING_GOES_HERE
  Mux : xbsIndex_r4/Mux  inputs=2  en=off  latency=1  precision=Full  arith_type=Unsigned  n_bits=16  bin_pt=14  quantization=Truncate  overflow=Wrap  dbl_ovrd=off  block_type=mux
      settings: block_version=8.2.02
  Mux1 : xbsIndex_r4/Mux  inputs=2  en=off  latency=1  precision=Full  arith_type=Unsigned  n_bits=16  bin_pt=14  quantization=Truncate  overflow=Wrap  dbl_ovrd=off  block_type=mux
      settings: block_version=8.2.02
  Posedge2 : Subsystem
  Register : xbsIndex_r4/Register  init=0  rst=off  en=off  dbl_ovrd=off  block_type=register
      settings: block_version=10.1
  Register1 : xbsIndex_r4/Register  init=0  rst=off  en=off  dbl_ovrd=off  block_type=register
      settings: block_version=10.1
  Register2 : xbsIndex_r4/Register  init=0  rst=off  en=off  dbl_ovrd=off  block_type=register
      settings: block_version=10.1
  Relational : xbsIndex_r4/Relational  mode=a>b  en=off  latency=0  dbl_ovrd=off  block_type=relational
      settings: block_version=8.2.02
  Rx_debug_antSel : xbsIndex_r4/Gateway Out  hdl_port=on  timing_constraint=None  locs_specified=off  LOCs={}  block_type=gatewayout
      settings: infoedit="Gateway out block.  Converts Xilinx fixed point inputs into ouputs of type Simulink integer, double, or fixed point.<P><P>Hardware notes:  In hardware these blocks become top level output ports or are discarded, depending on how they are configured."  xl_area=[0 0 0 0 1 0 0]  block_version=VER_STRING_GOES_HERE
  Up Sample : xbsIndex_r4/Up Sample  sample_ratio=4  copy_samples=on  dbl_ovrd=off  block_type=usamp
      settings: infoedit="Up samples input data.  Inserted values  can be zeros or copies of the most recent input sample.<P><P>Hardware notes: No hardware is needed if inserted values are copies of the input sample; otherwise, a mux and single bit flip-flop are used."  block_version=VER_STRING_GOES_HERE
  Up Sample1 : xbsIndex_r4/Up Sample  sample_ratio=4  copy_samples=on  dbl_ovrd=off  block_type=usamp
      settings: infoedit="Up samples input data.  Inserted values  can be zeros or copies of the most recent input sample.<P><P>Hardware notes: No hardware is needed if inserted values are copies of the input sample; otherwise, a mux and single bit flip-flop are used."  block_version=VER_STRING_GOES_HERE
  Up Sample2 : xbsIndex_r4/Up Sample  sample_ratio=4  copy_samples=on  dbl_ovrd=off  block_type=usamp
      settings: infoedit="Up samples input data.  Inserted values  can be zeros or copies of the most recent input sample.<P><P>Hardware notes: No hardware is needed if inserted values are copies of the input sample; otherwise, a mux and single bit flip-flop are used."  block_version=VER_STRING_GOES_HERE
  A I : Outport  OutDataType=sfix(16)  OutScaling=2^0
  A Q : Outport  Port=2  OutDataType=sfix(16)  OutScaling=2^0
  B I : Outport  Port=3  OutDataType=sfix(16)  OutScaling=2^0
  B Q : Outport  Port=4  OutDataType=sfix(16)  OutScaling=2^0
  tag scope: RxReg_RxGains=global, RxReg_RxGainsEn=global
  wires:
  AntB_I/1 -> Mux/3, Register2/1
  AntB_Q/1 -> Mux1/3, Register1/1
  AntA_I/1 -> Mux/2
  AntA_Q/1 -> Mux1/2
  Mux/1 -> A I/1
  Mux1/1 -> A Q/1
  PktDetReset/1 -> Up Sample1/1, AntB_Gains/1(Rst), AntA_Gains/1(Rst)
  AntA_Gains/2(GainVec) -> Relational/1, Concat2/3
  AntB_Gains/2(GainVec) -> Relational/2, Concat2/1
  Relational/1 -> Ant Sel Logic/1(G(B) < G(A))
  Ant Sel Logic/1(AntSel) -> Mux/1, Mux1/1, Up Sample2/1
  Up Sample2/1 -> Register/1
  Register2/1 -> B I/1
  Register1/1 -> B Q/1
  AntB_Gains/1(Vout) -> Ant Sel Logic/2(B_Vin)
  Concat2/1 -> Up Sample/1
  Up Sample/1 -> [RxReg_RxGains]
  Posedge2/1(Q) -> [RxReg_RxGainsEn]
  Constant6/1 -> Concat2/2
  Register/1 -> Rx_debug_antSel/1
  Up Sample1/1 -> Posedge2/1(D)

== system ofdm_txrx_mimo/OFDM Rx MIMO/Coarse Freq Correction/A*B ==
  Re[A] : Inport  OutDataType=sfix(16)  OutScaling=2^0
  Im[A] : Inport  Port=2  OutDataType=sfix(16)  OutScaling=2^0
  Im[B] : Inport  Port=3  OutDataType=sfix(16)  OutScaling=2^0
  Re[B] : Inport  Port=4  OutDataType=sfix(16)  OutScaling=2^0
  Add1 : xbsIndex_r4/AddSub  mode=Addition  use_carryin=off  use_carryout=off  en=off  latency=0  precision=User Defined  arith_type="Signed  (2's comp)"  n_bits=17  bin_pt=15  quantization=Truncate  overflow=Flag as error  dbl_ovrd=off  use_behavioral_HDL=on  pipelined=off  use_rpm=on  hw_selection=Fabric  block_type=addsub
      settings: xl_area=[9 0 0 16 0 0 0]  block_version=VER_STRING_GOES_HERE
  Counter : xbsIndex_r4/Counter  Orientation=down  cnt_type=Free Running  cnt_to=4  operation=Up  start_count=0  cnt_by_val=1  arith_type=Unsigned  n_bits=2  bin_pt=0  load_pin=off  rst=off  en=off  explicit_period=on  period=1  dbl_ovrd=off  use_behavioral_HDL=off  use_rpm=on  implementation=Fabric  block_type=counter
      settings: xl_area=[3 3 0 4 0 0 0]  block_version=VER_STRING_GOES_HERE
  Down Sample3 : xbsIndex_r4/Down Sample  sample_ratio=4  sample_phase=First Value of Frame  en=off  latency=1  dbl_ovrd=off  block_type=dsamp
      settings: xl_area=[9 17 0 0 0 0 0]  block_version=VER_STRING_GOES_HERE
  Down Sample4 : xbsIndex_r4/Down Sample  sample_ratio=4  sample_phase=First Value of Frame  en=off  latency=1  dbl_ovrd=off  block_type=dsamp
      settings: xl_area=[9 17 0 0 0 0 0]  block_version=VER_STRING_GOES_HERE
  Mult4 : xbsIndex_r4/Mult  precision=User Defined  arith_type="Signed  (2's comp)"  n_bits=17  bin_pt=16  quantization=Truncate  overflow=Flag as error  en=off  latency=1  dbl_ovrd=off  use_behavioral_HDL=off  use_embedded=on  opt=Speed  optimum_pipeline=off  pipeline=off  use_rpm=on  placement_style=Rectangular shape  block_type=mult
      settings: infoedit=Hardware notes: To use the internal pipeline stage of the dedicated multiplier you must select 'Pipeline for maximum performance'.  xl_area=[14 27 0 27 0 1 0]  block_version=VER_STRING_GOES_HERE
  Mux : xbsIndex_r4/Mux  inputs=4  en=off  latency=0  precision=Full  arith_type="Signed  (2's comp)"  n_bits=16  bin_pt=13  quantization=Truncate  overflow=Wrap  dbl_ovrd=off  block_type=mux
      settings: xl_area=[14 0 0 28 0 0 0]  block_version=VER_STRING_GOES_HERE
  Mux1 : xbsIndex_r4/Mux  inputs=4  en=off  latency=0  precision=Full  arith_type="Signed  (2's comp)"  n_bits=16  bin_pt=13  quantization=Truncate  overflow=Wrap  dbl_ovrd=off  block_type=mux
      settings: xl_area=[14 0 0 28 0 0 0]  block_version=VER_STRING_GOES_HERE
  Negate : xbsIndex_r4/Negate  precision=Full  arith_type="Signed  (2's comp)"  n_bits=14  bin_pt=13  quantization=Truncate  overflow=Flag as error  en=off  latency=0  dbl_ovrd=off  block_type=negate
      settings: xl_area=[7 0 0 14 0 0 0]  block_version=VER_STRING_GOES_HERE
  Register : xbsIndex_r4/Register  init=0  rst=off  en=off  dbl_ovrd=off  block_type=register
      settings: block_version=10.1.2
  Register1 : xbsIndex_r4/Register  init=0  rst=off  en=off  dbl_ovrd=off  block_type=register
      settings: block_version=10.1.2
  Register2 : xbsIndex_r4/Register  init=0  rst=off  en=off  dbl_ovrd=off  block_type=register
      settings: block_version=10.1.2
  Slice : xbsIndex_r4/Slice  nbits=2  boolean_output=off  mode=Lower Bit Location + Width  bit1=0  base1=MSB of Input  bit0=0  base0=LSB of Input  dbl_ovrd=off  block_type=slice
      settings: infoedit="Extracts a given range of bits from each input sample and presents it at the output.  The output type is ordinarily unsigned with binary point at zero, but can be Boolean when the slice is one bit wide.<P><P>Hardware notes: In hardware this block costs nothing."  block_version=VER_STRING_GOES_HERE
  Up Sample{1..4} (4 alike) : xbsIndex_r4/Up Sample  sample_ratio=4  copy_samples=on  dbl_ovrd=off  block_type=usamp
      settings: infoedit="Up samples input data.  Inserted values  can be zeros or copies of the most recent input sample.<P><P>Hardware notes: No hardware is needed if inserted values are copies of the input sample; otherwise, a mux and single bit flip-flop are used."  xl_area=[0 0 0 0 0 0 0]  block_version=VER_STRING_GOES_HERE
  Re : Outport  OutDataType=sfix(16)  OutScaling=2^0
  Im1 : Outport  Port=2  OutDataType=sfix(16)  OutScaling=2^0
  wires:
  Slice/1 -> Mux/1, Mux1/1
  Counter/1 -> Slice/1
  Register2/1 -> Add1/2
  Mult4/1 -> Add1/1, Register2/1
  Up Sample1/1 -> Mux1/5, Negate/1
  Up Sample4/1 -> Mux/4, Mux/3
  Up Sample2/1 -> Mux/2, Mux/5
  Up Sample3/1 -> Mux1/4, Mux1/2
  Re[A]/1 -> Up Sample3/1
  Re[B]/1 -> Up Sample2/1
  Im[A]/1 -> Up Sample1/1
  Im[B]/1 -> Up Sample4/1
  Mux1/1 -> Mult4/1
  Mux/1 -> Mult4/2
  Register/1 -> Register1/1
  Down Sample4/1 -> Im1/1
  Down Sample3/1 -> Re/1
  Add1/1 -> Down Sample4/1, Register/1
  Negate/1 -> Mux1/3
  Register1/1 -> Down Sample3/1

== system ofdm_txrx_mimo/OFDM Rx MIMO/Coarse Freq Correction/A*B1 ==
  Re[B] : Inport  OutDataType=sfix(16)  OutScaling=2^0
  Im[B] : Inport  Port=2  OutDataType=sfix(16)  OutScaling=2^0
  Re[A] : Inport  Port=3  OutDataType=sfix(16)  OutScaling=2^0
  Im[A] : Inport  Port=4  OutDataType=sfix(16)  OutScaling=2^0
  Add1 : xbsIndex_r4/AddSub  mode=Addition  use_carryin=off  use_carryout=off  en=off  latency=0  precision=User Defined  arith_type="Signed  (2's comp)"  n_bits=17  bin_pt=15  quantization=Truncate  overflow=Flag as error  dbl_ovrd=off  use_behavioral_HDL=on  pipelined=off  use_rpm=on  hw_selection=Fabric  block_type=addsub
      settings: xl_area=[9 0 0 16 0 0 0]  block_version=VER_STRING_GOES_HERE
  Counter : xbsIndex_r4/Counter  Orientation=down  cnt_type=Free Running  cnt_to=4  operation=Up  start_count=0  cnt_by_val=1  arith_type=Unsigned  n_bits=2  bin_pt=0  load_pin=off  rst=off  en=off  explicit_period=on  period=1  dbl_ovrd=off  use_behavioral_HDL=off  use_rpm=on  implementation=Fabric  block_type=counter
      settings: xl_area=[3 3 0 4 0 0 0]  block_version=VER_STRING_GOES_HERE
  Delay1 : xbsIndex_r4/Delay  en=off  latency=1  dbl_ovrd=off  reg_retiming=off  block_type=delay
      settings: infoedit=Hardware notes: A delay line is a chain, each link of which is an SRL16 followed by a flip-flop. If register retiming is enabled, the delay line is a chain of flip-flops.  xl_area=[8 16 0 0 0 0 0]  block_version=VER_STRING_GOES_HERE
  Delay2 : xbsIndex_r4/Delay  en=off  latency=2  dbl_ovrd=off  reg_retiming=off  block_type=delay
      settings: infoedit=Hardware notes: A delay line is a chain, each link of which is an SRL16 followed by a flip-flop. If register retiming is enabled, the delay line is a chain of flip-flops.  xl_area=[9 17 0 17 0 0 0]  block_version=VER_STRING_GOES_HERE
  Down Sample3 : xbsIndex_r4/Down Sample  sample_ratio=4  sample_phase=First Value of Frame  en=off  latency=1  dbl_ovrd=off  block_type=dsamp
      settings: xl_area=[9 17 0 0 0 0 0]  block_version=VER_STRING_GOES_HERE
  Down Sample4 : xbsIndex_r4/Down Sample  sample_ratio=4  sample_phase=First Value of Frame  en=off  latency=1  dbl_ovrd=off  block_type=dsamp
      settings: xl_area=[9 17 0 0 0 0 0]  block_version=VER_STRING_GOES_HERE
  Mult4 : xbsIndex_r4/Mult  precision=User Defined  arith_type="Signed  (2's comp)"  n_bits=16  bin_pt=15  quantization=Truncate  overflow=Flag as error  en=off  latency=1  dbl_ovrd=off  use_behavioral_HDL=off  use_embedded=on  opt=Speed  optimum_pipeline=off  pipeline=off  use_rpm=on  placement_style=Rectangular shape  block_type=mult
      settings: infoedit=Hardware notes: To use the internal pipeline stage of the dedicated multiplier you must select 'Pipeline for maximum performance'.  xl_area=[14 27 0 27 0 1 0]  block_version=VER_STRING_GOES_HERE
  Mux : xbsIndex_r4/Mux  inputs=4  en=off  latency=0  precision=Full  arith_type="Signed  (2's comp)"  n_bits=16  bin_pt=13  quantization=Truncate  overflow=Wrap  dbl_ovrd=off  block_type=mux
      settings: xl_area=[14 0 0 28 0 0 0]  block_version=VER_STRING_GOES_HERE
  Mux1 : xbsIndex_r4/Mux  inputs=4  en=off  latency=0  precision=Full  arith_type="Signed  (2's comp)"  n_bits=16  bin_pt=13  quantization=Truncate  overflow=Wrap  dbl_ovrd=off  block_type=mux
      settings: xl_area=[14 0 0 28 0 0 0]  block_version=VER_STRING_GOES_HERE
  Negate : xbsIndex_r4/Negate  precision=Full  arith_type="Signed  (2's comp)"  n_bits=32  bin_pt=30  quantization=Truncate  overflow=Flag as error  en=off  latency=0  dbl_ovrd=off  block_type=negate
      settings: xl_area=[7 0 0 14 0 0 0]  block_version=VER_STRING_GOES_HERE
  Slice : xbsIndex_r4/Slice  nbits=2  boolean_output=off  mode=Lower Bit Location + Width  bit1=0  base1=MSB of Input  bit0=0  base0=LSB of Input  dbl_ovrd=off  block_type=slice
      settings: infoedit="Extracts a given range of bits from each input sample and presents it at the output.  The output type is ordinarily unsigned with binary point at zero, but can be Boolean when the slice is one bit wide.<P><P>Hardware notes: In hardware this block costs nothing."  block_version=VER_STRING_GOES_HERE
  Up Sample{1..4} (4 alike) : xbsIndex_r4/Up Sample  sample_ratio=4  copy_samples=on  dbl_ovrd=off  block_type=usamp
      settings: infoedit="Up samples input data.  Inserted values  can be zeros or copies of the most recent input sample.<P><P>Hardware notes: No hardware is needed if inserted values are copies of the input sample; otherwise, a mux and single bit flip-flop are used."  xl_area=[0 0 0 0 0 0 0]  block_version=VER_STRING_GOES_HERE
  Re : Outport  OutDataType=sfix(16)  OutScaling=2^0
  Im1 : Outport  Port=2  OutDataType=sfix(16)  OutScaling=2^0
  wires:
  Add1/1 -> Delay2/1, Down Sample4/1
  Down Sample3/1 -> Re/1
  Down Sample4/1 -> Im1/1
  Delay2/1 -> Down Sample3/1
  Mux/1 -> Mult4/2
  Mux1/1 -> Mult4/1
  Im[B]/1 -> Up Sample4/1
  Im[A]/1 -> Up Sample1/1
  Re[B]/1 -> Up Sample2/1
  Re[A]/1 -> Up Sample3/1
  Up Sample3/1 -> Mux1/2, Mux1/4
  Up Sample2/1 -> Mux/5, Mux/2
  Up Sample4/1 -> Mux/3, Mux/4
  Up Sample1/1 -> Mux1/5, Negate/1
  Mult4/1 -> Add1/1, Delay1/1
  Delay1/1 -> Add1/2
  Counter/1 -> Slice/1
  Slice/1 -> Mux1/1, Mux/1
  Negate/1 -> Mux1/3
